simulink model "x64_32s_001"  (Simulink 7.4)
settings: Solver=ode45  StartTime=0.0  StopTime=100000  RelTol=1e-3
undefined symbols (not defined in the file): gcbh, ROACH, x64_32s_001_ct_sel_reg_user_data_out, x64_32s_001_inst_seng_id0_user_data_in, x64_32s_001_qdr_in_cnt_user_data_in, x64_32s_001_status_user_data_in, x64_32s_001_xaui_cnt0_user_data_in, x64_32s_001_xaui_error_cnt0_user_data_in, x64_32s_001_xauibuf_out_cnt_user_data_in, x64_32s_001_xauibuf_window_cnt_user_data_in, xlBlockLoadCallback
libraries: xbsIndex_r4 x7380, casper_library_misc x261, casper_library_delays x16, xps_library x6, ox_lib x1
(part 7 of 91)

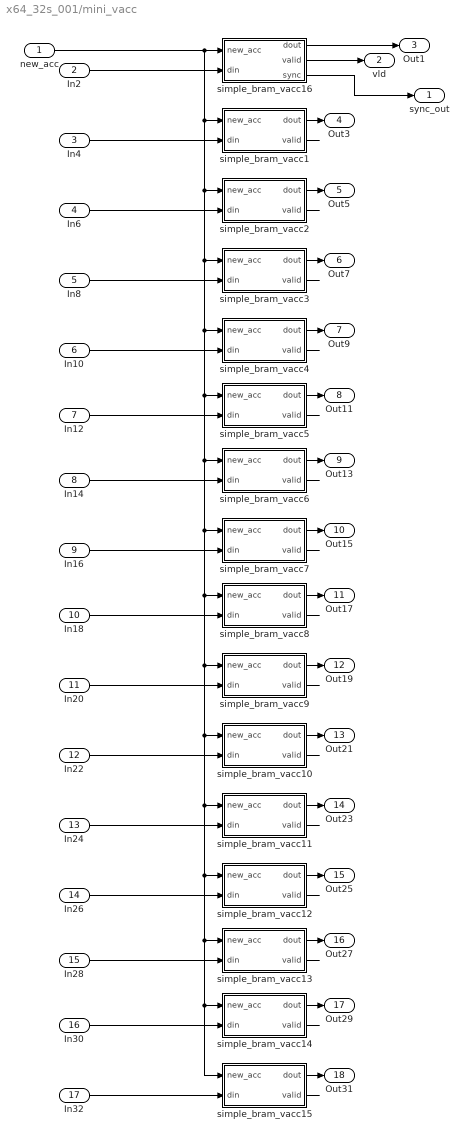
== system x64_32s_001/mini_vacc ==
  new_acc : Inport
  In2 : Inport  Port=2
  In4 : Inport  Port=3
  In6 : Inport  Port=4
  In8 : Inport  Port=5
  In10 : Inport  Port=6
  In12 : Inport  Port=7
  In14 : Inport  Port=8
  In16 : Inport  Port=9
  In18 : Inport  Port=10
  In20 : Inport  Port=11
  In22 : Inport  Port=12
  In24 : Inport  Port=13
  In26 : Inport  Port=14
  In28 : Inport  Port=15
  In30 : Inport  Port=16
  In32 : Inport  Port=17
  simple_bram_vacc1 : Subsystem  AncestorBlock=ox_lib/simple_bram_vacc  mask(simple_bram_vacc){vec_len=8, arith_type=Unsigned, n_bits=32, bin_pt=0, acc_len=128}
      mask prompts: vec_len "Vector length:"; arith_type "Output type:"; n_bits "Bit width (output):"; bin_pt "Binary point (output):"; acc_len "Accumulation length?"
      mask help:
      | This simple vector accumulator is based on a shift register. It outputs its accumulated vector as soon as it is calculated.
      |
      | (I.e, it does not require a further new acc pulse, and therefore won't break if there are periods of invalid data)
      mask init:
      | simple_bram_vacc_init(gcb, ...
      |     'vec_len', vec_len, ...
      |     'arith_type', arith_type, ...
      |     'n_bits', n_bits, ...
      |     'bin_pt', bin_pt);
      | 
      | fmtstr = sprintf('Acc len: %d', acc_len);
      | set_param(gcb, 'AttributesFormatString', fmtstr);
      settings: AttributesFormatString=Acc len: 128
      mask StyleString: edit,popup(Signed|Unsigned),edit,edit,edit
  simple_bram_vacc10 : Subsystem  AncestorBlock=ox_lib/simple_bram_vacc  mask(simple_bram_vacc){vec_len=8, arith_type=Unsigned, n_bits=32, bin_pt=0, acc_len=128}
      mask prompts: vec_len "Vector length:"; arith_type "Output type:"; n_bits "Bit width (output):"; bin_pt "Binary point (output):"; acc_len "Accumulation length?"
      mask help:
      | This simple vector accumulator is based on a shift register. It outputs its accumulated vector as soon as it is calculated.
      |
      | (I.e, it does not require a further new acc pulse, and therefore won't break if there are periods of invalid data)
      mask init: same as x64_32s_001/mini_vacc/simple_bram_vacc1
      settings: AttributesFormatString=Acc len: 128
      mask StyleString: edit,popup(Signed|Unsigned),edit,edit,edit
  simple_bram_vacc11 : Subsystem  AncestorBlock=ox_lib/simple_bram_vacc  mask(simple_bram_vacc){vec_len=8, arith_type=Unsigned, n_bits=32, bin_pt=0, acc_len=128}
      mask prompts: vec_len "Vector length:"; arith_type "Output type:"; n_bits "Bit width (output):"; bin_pt "Binary point (output):"; acc_len "Accumulation length?"
      mask help:
      | This simple vector accumulator is based on a shift register. It outputs its accumulated vector as soon as it is calculated.
      |
      | (I.e, it does not require a further new acc pulse, and therefore won't break if there are periods of invalid data)
      mask init: same as x64_32s_001/mini_vacc/simple_bram_vacc1
      settings: AttributesFormatString=Acc len: 128
      mask StyleString: edit,popup(Signed|Unsigned),edit,edit,edit
  simple_bram_vacc12 : Subsystem  AncestorBlock=ox_lib/simple_bram_vacc  mask(simple_bram_vacc){vec_len=8, arith_type=Unsigned, n_bits=32, bin_pt=0, acc_len=128}
      mask prompts: vec_len "Vector length:"; arith_type "Output type:"; n_bits "Bit width (output):"; bin_pt "Binary point (output):"; acc_len "Accumulation length?"
      mask help:
      | This simple vector accumulator is based on a shift register. It outputs its accumulated vector as soon as it is calculated.
      |
      | (I.e, it does not require a further new acc pulse, and therefore won't break if there are periods of invalid data)
      mask init: same as x64_32s_001/mini_vacc/simple_bram_vacc1
      settings: AttributesFormatString=Acc len: 128
      mask StyleString: edit,popup(Signed|Unsigned),edit,edit,edit
  simple_bram_vacc13 : Subsystem  AncestorBlock=ox_lib/simple_bram_vacc  mask(simple_bram_vacc){vec_len=8, arith_type=Unsigned, n_bits=32, bin_pt=0, acc_len=128}
      mask prompts: vec_len "Vector length:"; arith_type "Output type:"; n_bits "Bit width (output):"; bin_pt "Binary point (output):"; acc_len "Accumulation length?"
      mask help:
      | This simple vector accumulator is based on a shift register. It outputs its accumulated vector as soon as it is calculated.
      |
      | (I.e, it does not require a further new acc pulse, and therefore won't break if there are periods of invalid data)
      mask init: same as x64_32s_001/mini_vacc/simple_bram_vacc1
      settings: AttributesFormatString=Acc len: 128
      mask StyleString: edit,popup(Signed|Unsigned),edit,edit,edit
  simple_bram_vacc14 : Subsystem  AncestorBlock=ox_lib/simple_bram_vacc  mask(simple_bram_vacc){vec_len=8, arith_type=Unsigned, n_bits=32, bin_pt=0, acc_len=128}
      mask prompts: vec_len "Vector length:"; arith_type "Output type:"; n_bits "Bit width (output):"; bin_pt "Binary point (output):"; acc_len "Accumulation length?"
      mask help:
      | This simple vector accumulator is based on a shift register. It outputs its accumulated vector as soon as it is calculated.
      |
      | (I.e, it does not require a further new acc pulse, and therefore won't break if there are periods of invalid data)
      mask init: same as x64_32s_001/mini_vacc/simple_bram_vacc1
      settings: AttributesFormatString=Acc len: 128
      mask StyleString: edit,popup(Signed|Unsigned),edit,edit,edit
  simple_bram_vacc15 : Subsystem  AncestorBlock=ox_lib/simple_bram_vacc  mask(simple_bram_vacc){vec_len=8, arith_type=Unsigned, n_bits=32, bin_pt=0, acc_len=128}
      mask prompts: vec_len "Vector length:"; arith_type "Output type:"; n_bits "Bit width (output):"; bin_pt "Binary point (output):"; acc_len "Accumulation length?"
      mask help:
      | This simple vector accumulator is based on a shift register. It outputs its accumulated vector as soon as it is calculated.
      |
      | (I.e, it does not require a further new acc pulse, and therefore won't break if there are periods of invalid data)
      mask init: same as x64_32s_001/mini_vacc/simple_bram_vacc1
      settings: AttributesFormatString=Acc len: 128
      mask StyleString: edit,popup(Signed|Unsigned),edit,edit,edit
  simple_bram_vacc16 : Subsystem  AncestorBlock=ox_lib/simple_bram_vacc  mask(simple_bram_vacc){vec_len=8, arith_type=Unsigned, n_bits=32, bin_pt=0, acc_len=128}
      mask prompts: vec_len "Vector length:"; arith_type "Output type:"; n_bits "Bit width (output):"; bin_pt "Binary point (output):"; acc_len "Accumulation length?"
      mask help:
      | This simple vector accumulator is based on a shift register. It outputs its accumulated vector as soon as it is calculated.
      |
      | (I.e, it does not require a further new acc pulse, and therefore won't break if there are periods of invalid data)
      mask init: same as x64_32s_001/mini_vacc/simple_bram_vacc1
      settings: AttributesFormatString=Acc len: 128
      mask StyleString: edit,popup(Signed|Unsigned),edit,edit,edit
  simple_bram_vacc2 : Subsystem  AncestorBlock=ox_lib/simple_bram_vacc  mask(simple_bram_vacc){vec_len=8, arith_type=Unsigned, n_bits=32, bin_pt=0, acc_len=128}
      mask prompts: vec_len "Vector length:"; arith_type "Output type:"; n_bits "Bit width (output):"; bin_pt "Binary point (output):"; acc_len "Accumulation length?"
      mask help:
      | This simple vector accumulator is based on a shift register. It outputs its accumulated vector as soon as it is calculated.
      |
      | (I.e, it does not require a further new acc pulse, and therefore won't break if there are periods of invalid data)
      mask init: same as x64_32s_001/mini_vacc/simple_bram_vacc1
      settings: AttributesFormatString=Acc len: 128
      mask StyleString: edit,popup(Signed|Unsigned),edit,edit,edit
  simple_bram_vacc3 : Subsystem  AncestorBlock=ox_lib/simple_bram_vacc  mask(simple_bram_vacc){vec_len=8, arith_type=Unsigned, n_bits=32, bin_pt=0, acc_len=128}
      mask prompts: vec_len "Vector length:"; arith_type "Output type:"; n_bits "Bit width (output):"; bin_pt "Binary point (output):"; acc_len "Accumulation length?"
      mask help:
      | This simple vector accumulator is based on a shift register. It outputs its accumulated vector as soon as it is calculated.
      |
      | (I.e, it does not require a further new acc pulse, and therefore won't break if there are periods of invalid data)
      mask init: same as x64_32s_001/mini_vacc/simple_bram_vacc1
      settings: AttributesFormatString=Acc len: 128
      mask StyleString: edit,popup(Signed|Unsigned),edit,edit,edit
  simple_bram_vacc4 : Subsystem  AncestorBlock=ox_lib/simple_bram_vacc  mask(simple_bram_vacc){vec_len=8, arith_type=Unsigned, n_bits=32, bin_pt=0, acc_len=128}
      mask prompts: vec_len "Vector length:"; arith_type "Output type:"; n_bits "Bit width (output):"; bin_pt "Binary point (output):"; acc_len "Accumulation length?"
      mask help:
      | This simple vector accumulator is based on a shift register. It outputs its accumulated vector as soon as it is calculated.
      |
      | (I.e, it does not require a further new acc pulse, and therefore won't break if there are periods of invalid data)
      mask init: same as x64_32s_001/mini_vacc/simple_bram_vacc1
      settings: AttributesFormatString=Acc len: 128
      mask StyleString: edit,popup(Signed|Unsigned),edit,edit,edit
  simple_bram_vacc5 : Subsystem  AncestorBlock=ox_lib/simple_bram_vacc  mask(simple_bram_vacc){vec_len=8, arith_type=Unsigned, n_bits=32, bin_pt=0, acc_len=128}
      mask prompts: vec_len "Vector length:"; arith_type "Output type:"; n_bits "Bit width (output):"; bin_pt "Binary point (output):"; acc_len "Accumulation length?"
      mask help:
      | This simple vector accumulator is based on a shift register. It outputs its accumulated vector as soon as it is calculated.
      |
      | (I.e, it does not require a further new acc pulse, and therefore won't break if there are periods of invalid data)
      mask init: same as x64_32s_001/mini_vacc/simple_bram_vacc1
      settings: AttributesFormatString=Acc len: 128
      mask StyleString: edit,popup(Signed|Unsigned),edit,edit,edit
  simple_bram_vacc6 : Subsystem  AncestorBlock=ox_lib/simple_bram_vacc  mask(simple_bram_vacc){vec_len=8, arith_type=Unsigned, n_bits=32, bin_pt=0, acc_len=128}
      mask prompts: vec_len "Vector length:"; arith_type "Output type:"; n_bits "Bit width (output):"; bin_pt "Binary point (output):"; acc_len "Accumulation length?"
      mask help:
      | This simple vector accumulator is based on a shift register. It outputs its accumulated vector as soon as it is calculated.
      |
      | (I.e, it does not require a further new acc pulse, and therefore won't break if there are periods of invalid data)
      mask init: same as x64_32s_001/mini_vacc/simple_bram_vacc1
      settings: AttributesFormatString=Acc len: 128
      mask StyleString: edit,popup(Signed|Unsigned),edit,edit,edit
  simple_bram_vacc7 : Subsystem  AncestorBlock=ox_lib/simple_bram_vacc  mask(simple_bram_vacc){vec_len=8, arith_type=Unsigned, n_bits=32, bin_pt=0, acc_len=128}
      mask prompts: vec_len "Vector length:"; arith_type "Output type:"; n_bits "Bit width (output):"; bin_pt "Binary point (output):"; acc_len "Accumulation length?"
      mask help:
      | This simple vector accumulator is based on a shift register. It outputs its accumulated vector as soon as it is calculated.
      |
      | (I.e, it does not require a further new acc pulse, and therefore won't break if there are periods of invalid data)
      mask init: same as x64_32s_001/mini_vacc/simple_bram_vacc1
      settings: AttributesFormatString=Acc len: 128
      mask StyleString: edit,popup(Signed|Unsigned),edit,edit,edit
  simple_bram_vacc8 : Subsystem  AncestorBlock=ox_lib/simple_bram_vacc  mask(simple_bram_vacc){vec_len=8, arith_type=Unsigned, n_bits=32, bin_pt=0, acc_len=128}
      mask prompts: vec_len "Vector length:"; arith_type "Output type:"; n_bits "Bit width (output):"; bin_pt "Binary point (output):"; acc_len "Accumulation length?"
      mask help:
      | This simple vector accumulator is based on a shift register. It outputs its accumulated vector as soon as it is calculated.
      |
      | (I.e, it does not require a further new acc pulse, and therefore won't break if there are periods of invalid data)
      mask init: same as x64_32s_001/mini_vacc/simple_bram_vacc1
      settings: AttributesFormatString=Acc len: 128
      mask StyleString: edit,popup(Signed|Unsigned),edit,edit,edit
  simple_bram_vacc9 : Subsystem  AncestorBlock=ox_lib/simple_bram_vacc  mask(simple_bram_vacc){vec_len=8, arith_type=Unsigned, n_bits=32, bin_pt=0, acc_len=128}
      mask prompts: vec_len "Vector length:"; arith_type "Output type:"; n_bits "Bit width (output):"; bin_pt "Binary point (output):"; acc_len "Accumulation length?"
      mask help:
      | This simple vector accumulator is based on a shift register. It outputs its accumulated vector as soon as it is calculated.
      |
      | (I.e, it does not require a further new acc pulse, and therefore won't break if there are periods of invalid data)
      mask init: same as x64_32s_001/mini_vacc/simple_bram_vacc1
      settings: AttributesFormatString=Acc len: 128
      mask StyleString: edit,popup(Signed|Unsigned),edit,edit,edit
  sync_out : Outport
  vld : Outport  Port=2
  Out1 : Outport  Port=3
  Out3 : Outport  Port=4
  Out5 : Outport  Port=5
  Out7 : Outport  Port=6
  Out9 : Outport  Port=7
  Out11 : Outport  Port=8
  Out13 : Outport  Port=9
  Out15 : Outport  Port=10
  Out17 : Outport  Port=11
  Out19 : Outport  Port=12
  Out21 : Outport  Port=13
  Out23 : Outport  Port=14
  Out25 : Outport  Port=15
  Out27 : Outport  Port=16
  Out29 : Outport  Port=17
  Out31 : Outport  Port=18
  wires:
  simple_bram_vacc16/1(dout) -> Out1/1
  In2/1 -> simple_bram_vacc16/2(din)
  simple_bram_vacc1/1(dout) -> Out3/1
  In4/1 -> simple_bram_vacc1/2(din)
  simple_bram_vacc1/2(valid) -> ?
  simple_bram_vacc2/1(dout) -> Out5/1
  In6/1 -> simple_bram_vacc2/2(din)
  simple_bram_vacc2/2(valid) -> ?
  simple_bram_vacc3/1(dout) -> Out7/1
  In8/1 -> simple_bram_vacc3/2(din)
  simple_bram_vacc3/2(valid) -> ?
  simple_bram_vacc4/1(dout) -> Out9/1
  In10/1 -> simple_bram_vacc4/2(din)
  simple_bram_vacc4/2(valid) -> ?
  simple_bram_vacc5/1(dout) -> Out11/1
  In12/1 -> simple_bram_vacc5/2(din)
  simple_bram_vacc5/2(valid) -> ?
  simple_bram_vacc6/1(dout) -> Out13/1
  In14/1 -> simple_bram_vacc6/2(din)
  simple_bram_vacc6/2(valid) -> ?
  simple_bram_vacc7/1(dout) -> Out15/1
  In16/1 -> simple_bram_vacc7/2(din)
  simple_bram_vacc7/2(valid) -> ?
  simple_bram_vacc8/1(dout) -> Out17/1
  In18/1 -> simple_bram_vacc8/2(din)
  simple_bram_vacc8/2(valid) -> ?
  simple_bram_vacc9/1(dout) -> Out19/1
  In20/1 -> simple_bram_vacc9/2(din)
  simple_bram_vacc9/2(valid) -> ?
  simple_bram_vacc10/1(dout) -> Out21/1
  In22/1 -> simple_bram_vacc10/2(din)
  simple_bram_vacc10/2(valid) -> ?
  simple_bram_vacc11/1(dout) -> Out23/1
  In24/1 -> simple_bram_vacc11/2(din)
  simple_bram_vacc11/2(valid) -> ?
  simple_bram_vacc12/1(dout) -> Out25/1
  In26/1 -> simple_bram_vacc12/2(din)
  simple_bram_vacc12/2(valid) -> ?
  simple_bram_vacc13/1(dout) -> Out27/1
  In28/1 -> simple_bram_vacc13/2(din)
  simple_bram_vacc13/2(valid) -> ?
  simple_bram_vacc14/1(dout) -> Out29/1
  In30/1 -> simple_bram_vacc14/2(din)
  simple_bram_vacc14/2(valid) -> ?
  simple_bram_vacc15/1(dout) -> Out31/1
  In32/1 -> simple_bram_vacc15/2(din)
  simple_bram_vacc15/2(valid) -> ?
  simple_bram_vacc16/2(valid) -> vld/1
  new_acc/1 -> simple_bram_vacc14/1(new_acc), simple_bram_vacc15/1(new_acc), simple_bram_vacc13/1(new_acc), simple_bram_vacc12/1(new_acc), simple_bram_vacc11/1(new_acc), simple_bram_vacc10/1(new_acc), simple_bram_vacc9/1(new_acc), simple_bram_vacc8/1(new_acc), simple_bram_vacc7/1(new_acc), simple_bram_vacc6/1(new_acc), simple_bram_vacc5/1(new_acc), simple_bram_vacc4/1(new_acc), simple_bram_vacc3/1(new_acc), simple_bram_vacc2/1(new_acc), simple_bram_vacc1/1(new_acc), simple_bram_vacc16/1(new_acc)
  simple_bram_vacc16/3(sync) -> sync_out/1

== system x64_32s_001/mini_vacc_mux1 ==
  sync : Inport
  din : Inport  Port=2
  vld_in : Inport  Port=3
  Counter : xbsIndex_r4/Counter  cnt_type=Free Running  cnt_to=Inf  operation=Up  start_count=0  cnt_by_val=1  arith_type=Unsigned  n_bits=vec_len+4  bin_pt=0  load_pin=off  rst=on  en=off  explicit_period=on  period=1  dbl_ovrd=off  use_behavioral_HDL=off  implementation=Fabric  block_type=counter
      settings: block_version=11.4
  Delay : xbsIndex_r4/Delay  en=off  latency=2  dbl_ovrd=off  reg_retiming=off  block_type=delay
      settings: block_version=11.4
  Delay1 : xbsIndex_r4/Delay  en=off  latency=1  dbl_ovrd=off  reg_retiming=off  block_type=delay
      settings: block_version=11.4
  Delay2 : xbsIndex_r4/Delay  en=off  latency=1  dbl_ovrd=off  reg_retiming=off  block_type=delay
      settings: block_version=11.4
  Delay4 : xbsIndex_r4/Delay  en=off  latency=1  dbl_ovrd=off  reg_retiming=off  block_type=delay
      settings: block_version=11.4
  Mux : xbsIndex_r4/Mux  inputs=16  en=off  latency=2  precision=Full  arith_type=Unsigned  n_bits=16  bin_pt=14  quantization=Truncate  overflow=Wrap  dbl_ovrd=off  block_type=mux
      settings: block_version=11.4
  Single Port RAM{1..15} (15 alike) : xbsIndex_r4/Single Port RAM  depth=8  initVector=sin(pi*(0:15)/16)  distributed_mem=Distributed memory  write_mode=Read Before Write  rst=off  init_reg=0  en=off  latency=1  dbl_ovrd=off  optimize=Area  use_rpm=on  block_type=spram
  Slice : xbsIndex_r4/Slice  nbits=4  boolean_output=off  mode=Lower Bit Location + Width  bit1=0  base1=MSB of Input  bit0=vec_len  base0=LSB of Input  dbl_ovrd=off  block_type=slice
  Slice1 : xbsIndex_r4/Slice  nbits=vec_len  boolean_output=off  mode=Lower Bit Location + Width  bit1=0  base1=MSB of Input  bit0=0  base0=LSB of Input  dbl_ovrd=off  block_type=slice
  pulse_ext : casper_library_misc/pulse_ext  SystemSampleTime=-1  pulse_len=8*16
  uncram2 : Subsystem  AncestorBlock=gavrt_library/uncram  mask(uncram){num_slice=16, slice_width=32, bin_pt=0, arith_type=0}
      mask prompts: num_slice "Number of slices"; slice_width "Slice Width"; bin_pt "Output Binary Point"; arith_type "Output Arithmetic Type (0=Unsigned, 1=Signed)"
      mask help: Takes a concatenated input and slices it up into even pieces and reinterprets them as signed fixed point numbers with a given binary point.
      mask init:
      | uncram_init(gcb, ...
      |     'num_slice', num_slice, ...
      |     'slice_width', slice_width, ...
      |     'bin_pt', bin_pt, ...
      |     'arith_type', arith_type);
      settings: AttributesFormatString=UFix_32_0
  dout : Outport
  sync_out : Outport  Port=2
  vld : Outport  Port=3
  wires:
  Delay/1 -> sync_out/1, pulse_ext/1
  Slice/1 -> Delay2/1
  Counter/1 -> Slice/1, Slice1/1
  Mux/1 -> dout/1
  din/1 -> uncram2/1(In)
  sync/1 -> Counter/1, Delay1/1
  Delay2/1 -> Mux/1
  Delay1/1 -> Delay/1
  Single Port RAM1/1 -> Mux/3
  Single Port RAM2/1 -> Mux/4
  Single Port RAM3/1 -> Mux/5
  Single Port RAM4/1 -> Mux/6
  Single Port RAM5/1 -> Mux/7
  Single Port RAM6/1 -> Mux/8
  Single Port RAM7/1 -> Mux/9
  Single Port RAM8/1 -> Mux/10
  Single Port RAM9/1 -> Mux/11
  Single Port RAM10/1 -> Mux/12
  Single Port RAM11/1 -> Mux/13
  Single Port RAM12/1 -> Mux/14
  Single Port RAM13/1 -> Mux/15
  Single Port RAM14/1 -> Mux/16
  Single Port RAM15/1 -> Mux/17
  Slice1/1 -> Single Port RAM2/1, Single Port RAM3/1, Single Port RAM4/1, Single Port RAM5/1, Single Port RAM6/1, Single Port RAM7/1, Single Port RAM8/1, Single Port RAM9/1, Single Port RAM10/1, Single Port RAM11/1, Single Port RAM12/1, Single Port RAM13/1, Single Port RAM14/1, Single Port RAM15/1, Single Port RAM1/1
  vld_in/1 -> Single Port RAM1/3, Single Port RAM2/3, Single Port RAM3/3, Single Port RAM4/3, Single Port RAM5/3, Single Port RAM6/3, Single Port RAM7/3, Single Port RAM8/3, Single Port RAM9/3, Single Port RAM10/3, Single Port RAM11/3, Single Port RAM12/3, Single Port RAM13/3, Single Port RAM14/3, Single Port RAM15/3
  uncram2/1(Out1) -> Delay4/1
  uncram2/2(Out2) -> Single Port RAM1/2
  uncram2/3(Out3) -> Single Port RAM2/2
  uncram2/4(Out4) -> Single Port RAM3/2
  uncram2/5(Out5) -> Single Port RAM4/2
  uncram2/6(Out6) -> Single Port RAM5/2
  uncram2/7(Out7) -> Single Port RAM6/2
  uncram2/8(Out8) -> Single Port RAM7/2
  uncram2/9(Out9) -> Single Port RAM8/2
  uncram2/10(Out10) -> Single Port RAM9/2
  uncram2/11(Out11) -> Single Port RAM10/2
  uncram2/12(Out12) -> Single Port RAM11/2
  uncram2/13(Out13) -> Single Port RAM12/2
  uncram2/14(Out14) -> Single Port RAM13/2
  uncram2/15(Out15) -> Single Port RAM14/2
  uncram2/16(Out16) -> Single Port RAM15/2
  Delay4/1 -> Mux/2
  pulse_ext/1 -> vld/1

== system x64_32s_001/pow_sim_mux ==
  sync : Inport  OutDataType=fixdt(1, 16)  OutScaling=2^0
  din : Inport  Port=2  OutDataType=fixdt(1, 16)  OutScaling=2^0
  Convert3 : xbsIndex_r4/Convert  arith_type=Unsigned  n_bits=25  bin_pt=24  quantization="Round  (unbiased: Even Values)"  overflow=Wrap  latency=0  dbl_ovrd=off  pipeline=on  block_type=convert
      settings: block_version=11.4
  Counter : xbsIndex_r4/Counter  cnt_type=Free Running  cnt_to=Inf  operation=Up  start_count=0  cnt_by_val=1  arith_type=Unsigned  n_bits=3  bin_pt=0  load_pin=off  rst=on  en=off  explicit_period=on  period=1  dbl_ovrd=off  use_behavioral_HDL=off  implementation=Fabric  block_type=counter
      settings: block_version=11.4
  dout_sim : xbsIndex_r4/Simulation Multiplexer  sim_sel=1  hw_sel=2  block_type=simmux
      settings: infoedit="Distinguish input subsystems as \"simulation only\" and \"generation only\".  The input specified For Simulation will be used during Simulink simulation.  The input specified For Generation will be used during code generation.  This block will typically be used on the output of a HW Co-Simulation, Black Box, or ModelSim subsystem.<br><br>Hardware Notes: This block costs nothing."  block_version=11.4
  dout : Outport
  wires:
  Counter/1 -> Convert3/1
  din/1 -> dout_sim/2
  dout_sim/1 -> dout/1
  sync/1 -> Counter/1
  Convert3/1 -> dout_sim/1

== system x64_32s_001/pow_sim_mux1 ==
  sync : Inport  OutDataType=fixdt(1, 16)  OutScaling=2^0
  din : Inport  Port=2  OutDataType=fixdt(1, 16)  OutScaling=2^0
  Convert3 : xbsIndex_r4/Convert  arith_type=Unsigned  n_bits=25  bin_pt=24  quantization="Round  (unbiased: Even Values)"  overflow=Wrap  latency=0  dbl_ovrd=off  pipeline=on  block_type=convert
      settings: block_version=11.4
  Counter : xbsIndex_r4/Counter  cnt_type=Free Running  cnt_to=Inf  operation=Up  start_count=0  cnt_by_val=1  arith_type=Unsigned  n_bits=3  bin_pt=0  load_pin=off  rst=on  en=off  explicit_period=on  period=1  dbl_ovrd=off  use_behavioral_HDL=off  implementation=Fabric  block_type=counter
      settings: block_version=11.4
  dout_sim : xbsIndex_r4/Simulation Multiplexer  sim_sel=1  hw_sel=2  block_type=simmux
      settings: infoedit="Distinguish input subsystems as \"simulation only\" and \"generation only\".  The input specified For Simulation will be used during Simulink simulation.  The input specified For Generation will be used during code generation.  This block will typically be used on the output of a HW Co-Simulation, Black Box, or ModelSim subsystem.<br><br>Hardware Notes: This block costs nothing."  block_version=11.4
  dout : Outport
  wires:
  Convert3/1 -> dout_sim/1
  sync/1 -> Counter/1
  dout_sim/1 -> dout/1
  din/1 -> dout_sim/2
  Counter/1 -> Convert3/1

== system x64_32s_001/pow_sim_mux10 ==
  sync : Inport  OutDataType=fixdt(1, 16)  OutScaling=2^0
  din : Inport  Port=2  OutDataType=fixdt(1, 16)  OutScaling=2^0
  Convert3 : xbsIndex_r4/Convert  arith_type=Unsigned  n_bits=25  bin_pt=24  quantization="Round  (unbiased: Even Values)"  overflow=Wrap  latency=0  dbl_ovrd=off  pipeline=on  block_type=convert
      settings: block_version=11.4
  Counter : xbsIndex_r4/Counter  cnt_type=Free Running  cnt_to=Inf  operation=Up  start_count=0  cnt_by_val=1  arith_type=Unsigned  n_bits=3  bin_pt=0  load_pin=off  rst=on  en=off  explicit_period=on  period=1  dbl_ovrd=off  use_behavioral_HDL=off  implementation=Fabric  block_type=counter
      settings: block_version=11.4
  dout_sim : xbsIndex_r4/Simulation Multiplexer  sim_sel=1  hw_sel=2  block_type=simmux
      settings: infoedit="Distinguish input subsystems as \"simulation only\" and \"generation only\".  The input specified For Simulation will be used during Simulink simulation.  The input specified For Generation will be used during code generation.  This block will typically be used on the output of a HW Co-Simulation, Black Box, or ModelSim subsystem.<br><br>Hardware Notes: This block costs nothing."  block_version=11.4
  dout : Outport
  wires:
  Counter/1 -> Convert3/1
  din/1 -> dout_sim/2
  dout_sim/1 -> dout/1
  sync/1 -> Counter/1
  Convert3/1 -> dout_sim/1

== system x64_32s_001/pow_sim_mux11 ==
  sync : Inport  OutDataType=fixdt(1, 16)  OutScaling=2^0
  din : Inport  Port=2  OutDataType=fixdt(1, 16)  OutScaling=2^0
  Convert3 : xbsIndex_r4/Convert  arith_type=Unsigned  n_bits=25  bin_pt=24  quantization="Round  (unbiased: Even Values)"  overflow=Wrap  latency=0  dbl_ovrd=off  pipeline=on  block_type=convert
      settings: block_version=11.4
  Counter : xbsIndex_r4/Counter  cnt_type=Free Running  cnt_to=Inf  operation=Up  start_count=0  cnt_by_val=1  arith_type=Unsigned  n_bits=3  bin_pt=0  load_pin=off  rst=on  en=off  explicit_period=on  period=1  dbl_ovrd=off  use_behavioral_HDL=off  implementation=Fabric  block_type=counter
      settings: block_version=11.4
  dout_sim : xbsIndex_r4/Simulation Multiplexer  sim_sel=1  hw_sel=2  block_type=simmux
      settings: infoedit="Distinguish input subsystems as \"simulation only\" and \"generation only\".  The input specified For Simulation will be used during Simulink simulation.  The input specified For Generation will be used during code generation.  This block will typically be used on the output of a HW Co-Simulation, Black Box, or ModelSim subsystem.<br><br>Hardware Notes: This block costs nothing."  block_version=11.4
  dout : Outport
  wires:
  Convert3/1 -> dout_sim/1
  sync/1 -> Counter/1
  dout_sim/1 -> dout/1
  din/1 -> dout_sim/2
  Counter/1 -> Convert3/1

== system x64_32s_001/pow_sim_mux12 ==
  sync : Inport  OutDataType=fixdt(1, 16)  OutScaling=2^0
  din : Inport  Port=2  OutDataType=fixdt(1, 16)  OutScaling=2^0
  Convert3 : xbsIndex_r4/Convert  arith_type=Unsigned  n_bits=25  bin_pt=24  quantization="Round  (unbiased: Even Values)"  overflow=Wrap  latency=0  dbl_ovrd=off  pipeline=on  block_type=convert
      settings: block_version=11.4
  Counter : xbsIndex_r4/Counter  cnt_type=Free Running  cnt_to=Inf  operation=Up  start_count=0  cnt_by_val=1  arith_type=Unsigned  n_bits=3  bin_pt=0  load_pin=off  rst=on  en=off  explicit_period=on  period=1  dbl_ovrd=off  use_behavioral_HDL=off  implementation=Fabric  block_type=counter
      settings: block_version=11.4
  dout_sim : xbsIndex_r4/Simulation Multiplexer  sim_sel=1  hw_sel=2  block_type=simmux
      settings: infoedit="Distinguish input subsystems as \"simulation only\" and \"generation only\".  The input specified For Simulation will be used during Simulink simulation.  The input specified For Generation will be used during code generation.  This block will typically be used on the output of a HW Co-Simulation, Black Box, or ModelSim subsystem.<br><br>Hardware Notes: This block costs nothing."  block_version=11.4
  dout : Outport
  wires:
  Counter/1 -> Convert3/1
  din/1 -> dout_sim/2
  dout_sim/1 -> dout/1
  sync/1 -> Counter/1
  Convert3/1 -> dout_sim/1

== system x64_32s_001/pow_sim_mux13 ==
  sync : Inport  OutDataType=fixdt(1, 16)  OutScaling=2^0
  din : Inport  Port=2  OutDataType=fixdt(1, 16)  OutScaling=2^0
  Convert3 : xbsIndex_r4/Convert  arith_type=Unsigned  n_bits=25  bin_pt=24  quantization="Round  (unbiased: Even Values)"  overflow=Wrap  latency=0  dbl_ovrd=off  pipeline=on  block_type=convert
      settings: block_version=11.4
  Counter : xbsIndex_r4/Counter  cnt_type=Free Running  cnt_to=Inf  operation=Up  start_count=0  cnt_by_val=1  arith_type=Unsigned  n_bits=3  bin_pt=0  load_pin=off  rst=on  en=off  explicit_period=on  period=1  dbl_ovrd=off  use_behavioral_HDL=off  implementation=Fabric  block_type=counter
      settings: block_version=11.4
  dout_sim : xbsIndex_r4/Simulation Multiplexer  sim_sel=1  hw_sel=2  block_type=simmux
      settings: infoedit="Distinguish input subsystems as \"simulation only\" and \"generation only\".  The input specified For Simulation will be used during Simulink simulation.  The input specified For Generation will be used during code generation.  This block will typically be used on the output of a HW Co-Simulation, Black Box, or ModelSim subsystem.<br><br>Hardware Notes: This block costs nothing."  block_version=11.4
  dout : Outport
  wires:
  Convert3/1 -> dout_sim/1
  sync/1 -> Counter/1
  dout_sim/1 -> dout/1
  din/1 -> dout_sim/2
  Counter/1 -> Convert3/1

== system x64_32s_001/pow_sim_mux14 ==
  sync : Inport  OutDataType=fixdt(1, 16)  OutScaling=2^0
  din : Inport  Port=2  OutDataType=fixdt(1, 16)  OutScaling=2^0
  Convert3 : xbsIndex_r4/Convert  arith_type=Unsigned  n_bits=25  bin_pt=24  quantization="Round  (unbiased: Even Values)"  overflow=Wrap  latency=0  dbl_ovrd=off  pipeline=on  block_type=convert
      settings: block_version=11.4
  Counter : xbsIndex_r4/Counter  cnt_type=Free Running  cnt_to=Inf  operation=Up  start_count=0  cnt_by_val=1  arith_type=Unsigned  n_bits=3  bin_pt=0  load_pin=off  rst=on  en=off  explicit_period=on  period=1  dbl_ovrd=off  use_behavioral_HDL=off  implementation=Fabric  block_type=counter
      settings: block_version=11.4
  dout_sim : xbsIndex_r4/Simulation Multiplexer  sim_sel=1  hw_sel=2  block_type=simmux
      settings: infoedit="Distinguish input subsystems as \"simulation only\" and \"generation only\".  The input specified For Simulation will be used during Simulink simulation.  The input specified For Generation will be used during code generation.  This block will typically be used on the output of a HW Co-Simulation, Black Box, or ModelSim subsystem.<br><br>Hardware Notes: This block costs nothing."  block_version=11.4
  dout : Outport
  wires:
  Counter/1 -> Convert3/1
  din/1 -> dout_sim/2
  dout_sim/1 -> dout/1
  sync/1 -> Counter/1
  Convert3/1 -> dout_sim/1

== system x64_32s_001/pow_sim_mux15 ==
  sync : Inport  OutDataType=fixdt(1, 16)  OutScaling=2^0
  din : Inport  Port=2  OutDataType=fixdt(1, 16)  OutScaling=2^0
  Convert3 : xbsIndex_r4/Convert  arith_type=Unsigned  n_bits=25  bin_pt=24  quantization="Round  (unbiased: Even Values)"  overflow=Wrap  latency=0  dbl_ovrd=off  pipeline=on  block_type=convert
      settings: block_version=11.4
  Counter : xbsIndex_r4/Counter  cnt_type=Free Running  cnt_to=Inf  operation=Up  start_count=0  cnt_by_val=1  arith_type=Unsigned  n_bits=3  bin_pt=0  load_pin=off  rst=on  en=off  explicit_period=on  period=1  dbl_ovrd=off  use_behavioral_HDL=off  implementation=Fabric  block_type=counter
      settings: block_version=11.4
  dout_sim : xbsIndex_r4/Simulation Multiplexer  sim_sel=1  hw_sel=2  block_type=simmux
      settings: infoedit="Distinguish input subsystems as \"simulation only\" and \"generation only\".  The input specified For Simulation will be used during Simulink simulation.  The input specified For Generation will be used during code generation.  This block will typically be used on the output of a HW Co-Simulation, Black Box, or ModelSim subsystem.<br><br>Hardware Notes: This block costs nothing."  block_version=11.4
  dout : Outport
  wires:
  Convert3/1 -> dout_sim/1
  sync/1 -> Counter/1
  dout_sim/1 -> dout/1
  din/1 -> dout_sim/2
  Counter/1 -> Convert3/1

== system x64_32s_001/pow_sim_mux2 ==
  sync : Inport  OutDataType=fixdt(1, 16)  OutScaling=2^0
  din : Inport  Port=2  OutDataType=fixdt(1, 16)  OutScaling=2^0
  Convert3 : xbsIndex_r4/Convert  arith_type=Unsigned  n_bits=25  bin_pt=24  quantization="Round  (unbiased: Even Values)"  overflow=Wrap  latency=0  dbl_ovrd=off  pipeline=on  block_type=convert
      settings: block_version=11.4
  Counter : xbsIndex_r4/Counter  cnt_type=Free Running  cnt_to=Inf  operation=Up  start_count=0  cnt_by_val=1  arith_type=Unsigned  n_bits=3  bin_pt=0  load_pin=off  rst=on  en=off  explicit_period=on  period=1  dbl_ovrd=off  use_behavioral_HDL=off  implementation=Fabric  block_type=counter
      settings: block_version=11.4
  dout_sim : xbsIndex_r4/Simulation Multiplexer  sim_sel=1  hw_sel=2  block_type=simmux
      settings: infoedit="Distinguish input subsystems as \"simulation only\" and \"generation only\".  The input specified For Simulation will be used during Simulink simulation.  The input specified For Generation will be used during code generation.  This block will typically be used on the output of a HW Co-Simulation, Black Box, or ModelSim subsystem.<br><br>Hardware Notes: This block costs nothing."  block_version=11.4
  dout : Outport
  wires:
  Counter/1 -> Convert3/1
  din/1 -> dout_sim/2
  dout_sim/1 -> dout/1
  sync/1 -> Counter/1
  Convert3/1 -> dout_sim/1

== system x64_32s_001/pow_sim_mux3 ==
  sync : Inport  OutDataType=fixdt(1, 16)  OutScaling=2^0
  din : Inport  Port=2  OutDataType=fixdt(1, 16)  OutScaling=2^0
  Convert3 : xbsIndex_r4/Convert  arith_type=Unsigned  n_bits=25  bin_pt=24  quantization="Round  (unbiased: Even Values)"  overflow=Wrap  latency=0  dbl_ovrd=off  pipeline=on  block_type=convert
      settings: block_version=11.4
  Counter : xbsIndex_r4/Counter  cnt_type=Free Running  cnt_to=Inf  operation=Up  start_count=0  cnt_by_val=1  arith_type=Unsigned  n_bits=3  bin_pt=0  load_pin=off  rst=on  en=off  explicit_period=on  period=1  dbl_ovrd=off  use_behavioral_HDL=off  implementation=Fabric  block_type=counter
      settings: block_version=11.4
  dout_sim : xbsIndex_r4/Simulation Multiplexer  sim_sel=1  hw_sel=2  block_type=simmux
      settings: infoedit="Distinguish input subsystems as \"simulation only\" and \"generation only\".  The input specified For Simulation will be used during Simulink simulation.  The input specified For Generation will be used during code generation.  This block will typically be used on the output of a HW Co-Simulation, Black Box, or ModelSim subsystem.<br><br>Hardware Notes: This block costs nothing."  block_version=11.4
  dout : Outport
  wires:
  Convert3/1 -> dout_sim/1
  sync/1 -> Counter/1
  dout_sim/1 -> dout/1
  din/1 -> dout_sim/2
  Counter/1 -> Convert3/1

== system x64_32s_001/pow_sim_mux4 ==
  sync : Inport  OutDataType=fixdt(1, 16)  OutScaling=2^0
  din : Inport  Port=2  OutDataType=fixdt(1, 16)  OutScaling=2^0
  Convert3 : xbsIndex_r4/Convert  arith_type=Unsigned  n_bits=25  bin_pt=24  quantization="Round  (unbiased: Even Values)"  overflow=Wrap  latency=0  dbl_ovrd=off  pipeline=on  block_type=convert
      settings: block_version=11.4
  Counter : xbsIndex_r4/Counter  cnt_type=Free Running  cnt_to=Inf  operation=Up  start_count=0  cnt_by_val=1  arith_type=Unsigned  n_bits=3  bin_pt=0  load_pin=off  rst=on  en=off  explicit_period=on  period=1  dbl_ovrd=off  use_behavioral_HDL=off  implementation=Fabric  block_type=counter
      settings: block_version=11.4
  dout_sim : xbsIndex_r4/Simulation Multiplexer  sim_sel=1  hw_sel=2  block_type=simmux
      settings: infoedit="Distinguish input subsystems as \"simulation only\" and \"generation only\".  The input specified For Simulation will be used during Simulink simulation.  The input specified For Generation will be used during code generation.  This block will typically be used on the output of a HW Co-Simulation, Black Box, or ModelSim subsystem.<br><br>Hardware Notes: This block costs nothing."  block_version=11.4
  dout : Outport
  wires:
  Convert3/1 -> dout_sim/1
  sync/1 -> Counter/1
  dout_sim/1 -> dout/1
  din/1 -> dout_sim/2
  Counter/1 -> Convert3/1

== system x64_32s_001/pow_sim_mux5 ==
  sync : Inport  OutDataType=fixdt(1, 16)  OutScaling=2^0
  din : Inport  Port=2  OutDataType=fixdt(1, 16)  OutScaling=2^0
  Convert3 : xbsIndex_r4/Convert  arith_type=Unsigned  n_bits=25  bin_pt=24  quantization="Round  (unbiased: Even Values)"  overflow=Wrap  latency=0  dbl_ovrd=off  pipeline=on  block_type=convert
      settings: block_version=11.4
  Counter : xbsIndex_r4/Counter  cnt_type=Free Running  cnt_to=Inf  operation=Up  start_count=0  cnt_by_val=1  arith_type=Unsigned  n_bits=3  bin_pt=0  load_pin=off  rst=on  en=off  explicit_period=on  period=1  dbl_ovrd=off  use_behavioral_HDL=off  implementation=Fabric  block_type=counter
      settings: block_version=11.4
  dout_sim : xbsIndex_r4/Simulation Multiplexer  sim_sel=1  hw_sel=2  block_type=simmux
      settings: infoedit="Distinguish input subsystems as \"simulation only\" and \"generation only\".  The input specified For Simulation will be used during Simulink simulation.  The input specified For Generation will be used during code generation.  This block will typically be used on the output of a HW Co-Simulation, Black Box, or ModelSim subsystem.<br><br>Hardware Notes: This block costs nothing."  block_version=11.4
  dout : Outport
  wires:
  Counter/1 -> Convert3/1
  din/1 -> dout_sim/2
  dout_sim/1 -> dout/1
  sync/1 -> Counter/1
  Convert3/1 -> dout_sim/1

== system x64_32s_001/pow_sim_mux6 ==
  sync : Inport  OutDataType=fixdt(1, 16)  OutScaling=2^0
  din : Inport  Port=2  OutDataType=fixdt(1, 16)  OutScaling=2^0
  Convert3 : xbsIndex_r4/Convert  arith_type=Unsigned  n_bits=25  bin_pt=24  quantization="Round  (unbiased: Even Values)"  overflow=Wrap  latency=0  dbl_ovrd=off  pipeline=on  block_type=convert
      settings: block_version=11.4
  Counter : xbsIndex_r4/Counter  cnt_type=Free Running  cnt_to=Inf  operation=Up  start_count=0  cnt_by_val=1  arith_type=Unsigned  n_bits=3  bin_pt=0  load_pin=off  rst=on  en=off  explicit_period=on  period=1  dbl_ovrd=off  use_behavioral_HDL=off  implementation=Fabric  block_type=counter
      settings: block_version=11.4
  dout_sim : xbsIndex_r4/Simulation Multiplexer  sim_sel=1  hw_sel=2  block_type=simmux
      settings: infoedit="Distinguish input subsystems as \"simulation only\" and \"generation only\".  The input specified For Simulation will be used during Simulink simulation.  The input specified For Generation will be used during code generation.  This block will typically be used on the output of a HW Co-Simulation, Black Box, or ModelSim subsystem.<br><br>Hardware Notes: This block costs nothing."  block_version=11.4
  dout : Outport
  wires:
  Convert3/1 -> dout_sim/1
  sync/1 -> Counter/1
  dout_sim/1 -> dout/1
  din/1 -> dout_sim/2
  Counter/1 -> Convert3/1

== system x64_32s_001/pow_sim_mux7 ==
  sync : Inport  OutDataType=fixdt(1, 16)  OutScaling=2^0
  din : Inport  Port=2  OutDataType=fixdt(1, 16)  OutScaling=2^0
  Convert3 : xbsIndex_r4/Convert  arith_type=Unsigned  n_bits=25  bin_pt=24  quantization="Round  (unbiased: Even Values)"  overflow=Wrap  latency=0  dbl_ovrd=off  pipeline=on  block_type=convert
      settings: block_version=11.4
  Counter : xbsIndex_r4/Counter  cnt_type=Free Running  cnt_to=Inf  operation=Up  start_count=0  cnt_by_val=1  arith_type=Unsigned  n_bits=3  bin_pt=0  load_pin=off  rst=on  en=off  explicit_period=on  period=1  dbl_ovrd=off  use_behavioral_HDL=off  implementation=Fabric  block_type=counter
      settings: block_version=11.4
  dout_sim : xbsIndex_r4/Simulation Multiplexer  sim_sel=1  hw_sel=2  block_type=simmux
      settings: infoedit="Distinguish input subsystems as \"simulation only\" and \"generation only\".  The input specified For Simulation will be used during Simulink simulation.  The input specified For Generation will be used during code generation.  This block will typically be used on the output of a HW Co-Simulation, Black Box, or ModelSim subsystem.<br><br>Hardware Notes: This block costs nothing."  block_version=11.4
  dout : Outport
  wires:
  Counter/1 -> Convert3/1
  din/1 -> dout_sim/2
  dout_sim/1 -> dout/1
  sync/1 -> Counter/1
  Convert3/1 -> dout_sim/1

== system x64_32s_001/pow_sim_mux8 ==
  sync : Inport  OutDataType=fixdt(1, 16)  OutScaling=2^0
  din : Inport  Port=2  OutDataType=fixdt(1, 16)  OutScaling=2^0
  Convert3 : xbsIndex_r4/Convert  arith_type=Unsigned  n_bits=25  bin_pt=24  quantization="Round  (unbiased: Even Values)"  overflow=Wrap  latency=0  dbl_ovrd=off  pipeline=on  block_type=convert
      settings: block_version=11.4
  Counter : xbsIndex_r4/Counter  cnt_type=Free Running  cnt_to=Inf  operation=Up  start_count=0  cnt_by_val=1  arith_type=Unsigned  n_bits=3  bin_pt=0  load_pin=off  rst=on  en=off  explicit_period=on  period=1  dbl_ovrd=off  use_behavioral_HDL=off  implementation=Fabric  block_type=counter
      settings: block_version=11.4
  dout_sim : xbsIndex_r4/Simulation Multiplexer  sim_sel=1  hw_sel=2  block_type=simmux
      settings: infoedit="Distinguish input subsystems as \"simulation only\" and \"generation only\".  The input specified For Simulation will be used during Simulink simulation.  The input specified For Generation will be used during code generation.  This block will typically be used on the output of a HW Co-Simulation, Black Box, or ModelSim subsystem.<br><br>Hardware Notes: This block costs nothing."  block_version=11.4
  dout : Outport
  wires:
  Counter/1 -> Convert3/1
  din/1 -> dout_sim/2
  dout_sim/1 -> dout/1
  sync/1 -> Counter/1
  Convert3/1 -> dout_sim/1

== system x64_32s_001/pow_sim_mux9 ==
  sync : Inport  OutDataType=fixdt(1, 16)  OutScaling=2^0
  din : Inport  Port=2  OutDataType=fixdt(1, 16)  OutScaling=2^0
  Convert3 : xbsIndex_r4/Convert  arith_type=Unsigned  n_bits=25  bin_pt=24  quantization="Round  (unbiased: Even Values)"  overflow=Wrap  latency=0  dbl_ovrd=off  pipeline=on  block_type=convert
      settings: block_version=11.4
  Counter : xbsIndex_r4/Counter  cnt_type=Free Running  cnt_to=Inf  operation=Up  start_count=0  cnt_by_val=1  arith_type=Unsigned  n_bits=3  bin_pt=0  load_pin=off  rst=on  en=off  explicit_period=on  period=1  dbl_ovrd=off  use_behavioral_HDL=off  implementation=Fabric  block_type=counter
      settings: block_version=11.4
  dout_sim : xbsIndex_r4/Simulation Multiplexer  sim_sel=1  hw_sel=2  block_type=simmux
      settings: infoedit="Distinguish input subsystems as \"simulation only\" and \"generation only\".  The input specified For Simulation will be used during Simulink simulation.  The input specified For Generation will be used during code generation.  This block will typically be used on the output of a HW Co-Simulation, Black Box, or ModelSim subsystem.<br><br>Hardware Notes: This block costs nothing."  block_version=11.4
  dout : Outport
  wires:
  Convert3/1 -> dout_sim/1
  sync/1 -> Counter/1
  dout_sim/1 -> dout/1
  din/1 -> dout_sim/2
  Counter/1 -> Convert3/1

== system x64_32s_001/qdr_in_cnt ==
  reg_out : Inport  OutDataType=sfix(16)  OutScaling=2^0
  convert : xbsIndex_r4/Convert  arith_type=Unsigned  n_bits=32  bin_pt=0  quantization=Truncate  overflow=Wrap  latency=0  dbl_ovrd=off  pipeline=off  block_type=convert
      settings: block_version=11.4
  x64_32s_001_qdr_in_cnt_user_data_in : xbsIndex_r4/Gateway Out  hdl_port=on  timing_constraint=None  locs_specified=off  LOCs={}  block_type=gatewayout
      settings: infoedit="Gateway out block.  Converts Xilinx fixed point inputs into ouputs of type Simulink integer, double, or fixed point.<br><br>Hardware notes:  In hardware these blocks become top level output ports or are discarded, depending on how they are configured."  block_version=11.4
  sim_out : Outport  OutDataType=sfix(16)  OutScaling=2^0
  wires:
  reg_out/1 -> convert/1
  convert/1 -> x64_32s_001_qdr_in_cnt_user_data_in/1
  x64_32s_001_qdr_in_cnt_user_data_in/1 -> sim_out/1
